simulink model "siento3"  (Simulink 7.2)
settings: Solver=ode45  StartTime=0.0  StopTime=10.0  RelTol=1e-3
undefined symbols (not defined in the file or in its folder): ecgGen, emgGen, fdesignblkfcn, gcbh, scGen
libraries: dspsigops x684, dspbuff3 x3, dspsrcs4 x3
(part 1 of 5)
settings Model: Creator=rafa  LastModifiedBy=rafa

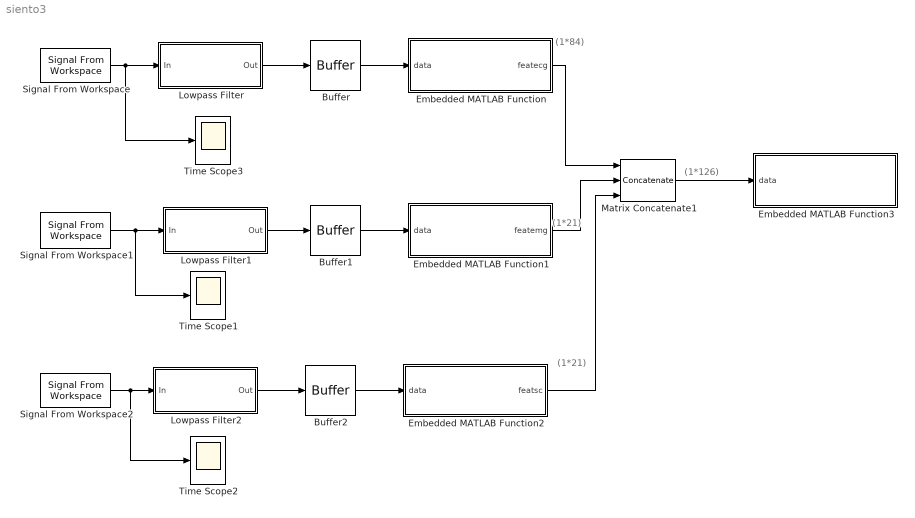
== system siento3 ==
  note: (1*84)
  note: (1*21)
  note: (1*21)
  note: (1*126)
  Buffer : dspbuff3/Buffer  N=1000  V=0  ic=0
  Buffer1 : dspbuff3/Buffer  N=1000  V=0  ic=0
  Buffer2 : dspbuff3/Buffer  N=1000  V=0  ic=0
  Embedded MATLAB Function : Stateflow chart
  Embedded MATLAB Function1 : Stateflow chart
  Embedded MATLAB Function2 : Stateflow chart
  Embedded MATLAB Function3 : Stateflow chart
  Lowpass Filter : Subsystem  DialogController=fdesignblkfcn  DialogControllerArgs=DataTag1  InitFcn=fdesignblkfcn(gcbh, {'InitFcn'});
      settings: CopyFcn=fdesignblkfcn(gcbh, {'CopyFcn'});
      mask Type: Lowpass Filter
      mask Description: Design a lowpass filter
      mask Help: helpview(mapfilelocation('dspblks'), 'dsplowpassfilter')
      mask Display: disp('Lowpass')
  Lowpass Filter1 : Subsystem  DialogController=fdesignblkfcn  DialogControllerArgs=DataTag4  InitFcn=fdesignblkfcn(gcbh, {'InitFcn'});
      settings: CopyFcn=fdesignblkfcn(gcbh, {'CopyFcn'});
      mask Type: Lowpass Filter
      mask Description: Design a lowpass filter
      mask Help: helpview(mapfilelocation('dspblks'), 'dsplowpassfilter')
      mask Display: disp('Lowpass')
  Lowpass Filter2 : Subsystem  DialogController=fdesignblkfcn  DialogControllerArgs=DataTag7  InitFcn=fdesignblkfcn(gcbh, {'InitFcn'});
      settings: CopyFcn=fdesignblkfcn(gcbh, {'CopyFcn'});
      mask Type: Lowpass Filter
      mask Description: Design a lowpass filter
      mask Help: helpview(mapfilelocation('dspblks'), 'dsplowpassfilter')
      mask Display: disp('Lowpass')
  Matrix Concatenate1 : Concatenate  NumInputs=3  Mode=Multidimensional array
  Signal From Workspace : dspsrcs4/Signal From Workspace  SystemSampleTime=-1  X=ecgGen(10)  Ts=0.01  nsamps=1  OutputAfterFinalValue=Cyclic repetition  ignoreOrWarnInputAndFrameLengths=off
  Signal From Workspace1 : dspsrcs4/Signal From Workspace  SystemSampleTime=-1  X=emgGen(10)  Ts=0.01  nsamps=1  OutputAfterFinalValue=Setting to zero  ignoreOrWarnInputAndFrameLengths=off
  Signal From Workspace2 : dspsrcs4/Signal From Workspace  SystemSampleTime=-1  X=scGen(10)  Ts=0.01  nsamps=1  OutputAfterFinalValue=Setting to zero  ignoreOrWarnInputAndFrameLengths=off
  Time Scope1 : Scope
      settings: DataFormat=Structure
  Time Scope2 : Scope
      settings: SaveName=ScopeData2  DataFormat=Structure
  Time Scope3 : Scope
      settings: DataFormat=Structure
  wires:
  Buffer/1 -> Embedded MATLAB Function/1(data)
  Embedded MATLAB Function/1(featecg) -> Matrix Concatenate1/1
  Buffer1/1 -> Embedded MATLAB Function1/1(data)
  Embedded MATLAB Function1/1(featemg) -> Matrix Concatenate1/2
  Buffer2/1 -> Embedded MATLAB Function2/1(data)
  Embedded MATLAB Function2/1(featsc) -> Matrix Concatenate1/3
  Matrix Concatenate1/1 -> Embedded MATLAB Function3/1(data)
  Signal From Workspace/1 -> Lowpass Filter/1(In), Time Scope3/1
  Lowpass Filter/1(Out) -> Buffer/1
  Signal From Workspace1/1 -> Time Scope1/1, Lowpass Filter1/1(In)
  Lowpass Filter1/1(Out) -> Buffer1/1
  Signal From Workspace2/1 -> Lowpass Filter2/1(In), Time Scope2/1
  Lowpass Filter2/1(Out) -> Buffer2/1

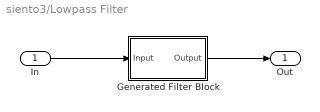
== system siento3/Lowpass Filter ==
  In : Inport  OutDataType=sfix(16)  OutScaling=2^0
  Generated Filter Block : Subsystem  Tag=FilterWizardSubSystem
      settings: ErrorFcn=dspFilterRealizedInBasicElemsAlgLoopErrFcnCallback
  Out : Outport  OutDataType=sfix(16)  OutScaling=2^0
  wires:
  In/1 -> Generated Filter Block/1(Input)
  Generated Filter Block/1(Output) -> Out/1

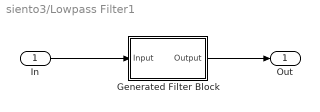
== system siento3/Lowpass Filter1 ==
  In : Inport  OutDataType=sfix(16)  OutScaling=2^0
  Generated Filter Block : Subsystem  Tag=FilterWizardSubSystem
      settings: ErrorFcn=dspFilterRealizedInBasicElemsAlgLoopErrFcnCallback
  Out : Outport  OutDataType=sfix(16)  OutScaling=2^0
  wires:
  In/1 -> Generated Filter Block/1(Input)
  Generated Filter Block/1(Output) -> Out/1

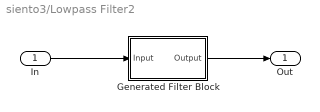
== system siento3/Lowpass Filter2 ==
  In : Inport  OutDataType=sfix(16)  OutScaling=2^0
  Generated Filter Block : Subsystem  Tag=FilterWizardSubSystem
      settings: ErrorFcn=dspFilterRealizedInBasicElemsAlgLoopErrFcnCallback
  Out : Outport  OutDataType=sfix(16)  OutScaling=2^0
  wires:
  In/1 -> Generated Filter Block/1(Input)
  Generated Filter Block/1(Output) -> Out/1
